simulink model "ofdm_txrx_mimo_2x"  (Simulink 6.6)
settings: Solver=VariableStepDiscrete  StartTime=0.0  StopTime=20e3  RelTol=1e-3
PreLoadFcn:
  | ofdm_rx_mimo_init
InitFcn:
  | ofdm_rx_mimo_init
StartFcn:
  | ofdm_rx_mimo_init
values from neighbouring files (symbols this model uses):
  BER_RAM_init_size = 2048    [ofdm_tx_mimo_init.m]
  CPLength = 16    [ofdm_tx_mimo_init.m]
  CRCPolynomial = hex2dec('04c11db7')    [ofdm_tx_mimo_init.m]
  freqOffset_track_filtCoef_i = hex2dec('2500')/2^32    [ofdm_rx_mimo_init.m]
  freqOffset_track_filtCoef_p = hex2dec('d0000')/2^32    [ofdm_rx_mimo_init.m]
  hi = zeros(1,length(cci))    [ofdm_rx_mimo_init.m]
  hr = zeros(1,length(ccr))    [ofdm_rx_mimo_init.m]
  max_CP_length = 16    [ofdm_tx_mimo_init.m]
  max_num_baseRateSymbols = 31    [ofdm_tx_mimo_init.m]
  max_num_subcarriers = 64    [ofdm_tx_mimo_init.m]
  max_num_trainingSymbols = 15    [ofdm_tx_mimo_init.m]
  max_numBytes = 16384    [ofdm_tx_mimo_init.m]
  max_OFDM_symbols = 2047    [ofdm_tx_mimo_init.m]
  modConstellation_qam16 = .75*[1 3 -1 -3]./3    [ofdm_tx_mimo_init.m]
  modConstellation_qam256 = 0.9375*[3 1 5 7 11 9 13 15 -3 -1 -5 -7 -11 -9 -13 -15]./15    [ofdm_tx_mimo_init.m]
  modConstellation_qam64 = 0.875*[3 1 5 7 -3 -1 -5 -7]./7    [ofdm_tx_mimo_init.m]
  modConstellation_qpsk = [1 -1]./sqrt(2)    [ofdm_tx_mimo_init.m]
  numBaseRateSymbols = 2    [ofdm_tx_mimo_init.m]
  numSubcarriers = 64    [ofdm_tx_mimo_init.m]
  numTrainingSymbols = 2    [ofdm_tx_mimo_init.m]
  pilot1_indicies = 7 + ( (64-7) * 2^16)    (= 3735559)    [ofdm_tx_mimo_init.m]
  pilot2_indicies = 21 + ( (64-21) * 2^16)    (= 2818069)    [ofdm_tx_mimo_init.m]
  pilotValue_neg = hex2dec('8000') + (2^16 * 0)    [ofdm_tx_mimo_init.m]
  pilotValue_pos = hex2dec('7FFF') + (2^16 * 0)    [ofdm_tx_mimo_init.m]
  pkt_crossCorr_thresh = 0.7    [ofdm_rx_mimo_init.m]
  pkt_energy_thresh = 0    [ofdm_rx_mimo_init.m]
  pkt_numPayloadBytes = 1400+42-6    (= 1436)    [ofdm_tx_mimo_init.m]
  pktByteNums = numHeaderBytes +  				byteIndex_numPayloadBytes(1)*2^8 +  				byteIndex_numPayloadBytes(2)*2^16 +  				byteIndex_simpleDynModMasks * 2^24    [ofdm_rx_mimo_init.m]
  PktDet_Delay = 26 + Symbol_Timing_Offset*2^7    (= 1050)    [ofdm_rx_mimo_init.m]
  preamble = 6*[0 shortsyms_10 longsyms_2]    [ofdm_tx_mimo_init.m]
  preamble_I = real(preamble)    [ofdm_tx_mimo_init.m]
  preamble_Q = imag(preamble)    [ofdm_tx_mimo_init.m]
  RAM_init_size = max_numBytes    (= 16384)    [ofdm_tx_mimo_init.m]
  reg_Interrupt_PktBuf_Control = reg_InterruptControl +  	1 * 2^16 +  	0 * 2^24    (= 65656)    [ofdm_rx_mimo_init.m]
  rx_controlBits = 1 * 2  		 + 1 * 4  		 + 0 * 8  		 + 1 * 16 * rx_SISO_Mode  		 + 1 * 32  		 + 1 * 64  		 + 0 * 128  		 + 1 * 256  		 + 0 * 512  		 + 1 * 1024  		 + 0 * 2048  		 + 1 * 4096  		 + 1 * 8192  		 + 0 * 16384  		 + 1 * 65536  		 + 1 * 131072  		 + 0 * 262144  		 + 1 * 524288    [ofdm_rx_mimo_init.m]
  Rx_PhaseNoiseTrack_K = 0.6094    [ofdm_rx_mimo_init.m]
  rx_postEq_scaling = round(rxScaling*2^11) * (1 + 2^16)    [ofdm_rx_mimo_init.m]
  RxDataScrambling_Seq = [40 198 78 63 82 173 102 245 48 111 172 115 147 230 216 93 72 65 62 2 205 242 122 90 128 83 105 97 73 10 5 252]    [ofdm_tx_mimo_init.m]
  simOnly_numSamples = length(preamble)+( (numSubcarriers+CPLength)*(numTrainingSymbols + numBaseRateSymbols + numFullRateSymbols/2) )    [ofdm_tx_mimo_init.m]
  train = [train train]    [ofdm_tx_mimo_init.m]
  tx_controlBits = (antB_preambleShift * 2^4) + (2^0 * tx_SISO_Mode) + 4*0 + 8*1    (= 73)    [ofdm_tx_mimo_init.m]
  TxDataScrambling_Seq = [40 198 78 63 82 173 102 245 48 111 172 115 147 230 216 93 72 65 62 2 205 242 122 90 128 83 105 97 73 10 5 252]    [ofdm_tx_mimo_init.m]
  txReg_symbolCounts = (2^16 * numFullRateSymbols) + (2^8 *numBaseRateSymbols ) + numTrainingSymbols    [ofdm_tx_mimo_init.m]
  TxRx_FFTScaling = bin2dec('010111') + (bin2dec('000101') * 2^6)    [ofdm_tx_mimo_init.m]
undefined symbols (not defined in the file or in its folder): AntA, AntB, BaseRate, CRC_table_gen, gcbh, intfilt, pktTiming_count_payloadStart, pktTiming_symbol_length, xlBlockLoadCallback, xlGetSimulinkPeriod
libraries: xbsIndex_r4 x3973, dspsigops x4, commchan3 x2, commrflib2 x2, dspsrcs4 x1
(part 20 of 38)

== system ofdm_txrx_mimo_2x/OFDM Tx MIMO/OutputBuffers/RAMs/Antenna A RAM ==
  I : Inport
  Q : Inport  Port=2
  We : Inport  Port=3
  Wr Addr : Inport  Port=4
  Rd Addr : Inport  Port=5
  OE# : Inport  Port=6
  Constant8 : xbsIndex_r4/Constant  arith_type=Boolean  const=0  n_bits=1  bin_pt=0  explicit_period=off  period=1  equ=P=C  opselect=C  inp2=PCIN>>17  opr=+  inp1=P  carry=CIN  dbl_ovrd=off  block_type=constant
      settings: block_version=VER_STRING_GOES_HERE
  Dual Port RAM : xbsIndex_r4/Dual Port RAM  depth=2*numSubcarriers  initVector=[repmat(0.25,1,64) repmat(0.5,1,64)]  distributed_mem=Block RAM  init_a=0  init_b=0  rst_a=off  rst_b=on  en_a=off  en_b=off  latency=1  write_mode_A=Read Before Write  write_mode_B=Read Before Write  dbl_ovrd=off  optimize=Area  use_rpm=off  block_type=dpram
      settings: xl_area=[0 0 1 0 0 0 0]  block_version=VER_STRING_GOES_HERE
  Dual Port RAM1 : xbsIndex_r4/Dual Port RAM  depth=2*numSubcarriers  initVector=[repmat(0.25,1,64) repmat(0.5,1,64)]  distributed_mem=Block RAM  init_a=0  init_b=0  rst_a=off  rst_b=on  en_a=off  en_b=off  latency=1  write_mode_A=Read Before Write  write_mode_B=Read Before Write  dbl_ovrd=off  optimize=Area  use_rpm=off  block_type=dpram
      settings: xl_area=[0 0 1 0 0 0 0]  block_version=VER_STRING_GOES_HERE
  " I" : Outport
  " Q" : Outport  Port=2
  wires:
  OE#/1 -> Dual Port RAM1/7, Dual Port RAM/7
  We/1 -> Dual Port RAM1/3, Dual Port RAM/3
  Q/1 -> Dual Port RAM1/2, Dual Port RAM1/5
  I/1 -> Dual Port RAM/2, Dual Port RAM/5
  Dual Port RAM/2 -> " I"/1
  Dual Port RAM1/2 -> " Q"/1
  Rd Addr/1 -> Dual Port RAM/4, Dual Port RAM1/4
  Constant8/1 -> Dual Port RAM1/6, Dual Port RAM/6
  Wr Addr/1 -> Dual Port RAM/1, Dual Port RAM1/1

== system ofdm_txrx_mimo_2x/OFDM Tx MIMO/OutputBuffers/RAMs/Antenna B RAM ==
  I : Inport
  Q : Inport  Port=2
  We : Inport  Port=3
  Wr Addr : Inport  Port=4
  Rd Addr : Inport  Port=5
  OE# : Inport  Port=6
  Constant8 : xbsIndex_r4/Constant  arith_type=Boolean  const=0  n_bits=1  bin_pt=0  explicit_period=off  period=1  equ=P=C  opselect=C  inp2=PCIN>>17  opr=+  inp1=P  carry=CIN  dbl_ovrd=off  block_type=constant
      settings: block_version=VER_STRING_GOES_HERE
  Dual Port RAM : xbsIndex_r4/Dual Port RAM  depth=2*numSubcarriers  initVector=-[repmat(0.25,1,64) repmat(0.5,1,64)]  distributed_mem=Block RAM  init_a=0  init_b=0  rst_a=off  rst_b=on  en_a=off  en_b=off  latency=1  write_mode_A=Read Before Write  write_mode_B=Read Before Write  dbl_ovrd=off  optimize=Area  use_rpm=off  block_type=dpram
      settings: xl_area=[0 0 1 0 0 0 0]  block_version=VER_STRING_GOES_HERE
  Dual Port RAM1 : xbsIndex_r4/Dual Port RAM  depth=2*numSubcarriers  initVector=-[repmat(0.25,1,64) repmat(0.5,1,64)]  distributed_mem=Block RAM  init_a=0  init_b=0  rst_a=off  rst_b=on  en_a=off  en_b=off  latency=1  write_mode_A=Read Before Write  write_mode_B=Read Before Write  dbl_ovrd=off  optimize=Area  use_rpm=off  block_type=dpram
      settings: xl_area=[0 0 1 0 0 0 0]  block_version=VER_STRING_GOES_HERE
  " I" : Outport
  " Q" : Outport  Port=2
  wires:
  Wr Addr/1 -> Dual Port RAM1/1, Dual Port RAM/1
  Constant8/1 -> Dual Port RAM/6, Dual Port RAM1/6
  Rd Addr/1 -> Dual Port RAM1/4, Dual Port RAM/4
  Dual Port RAM1/2 -> " Q"/1
  Dual Port RAM/2 -> " I"/1
  I/1 -> Dual Port RAM/5, Dual Port RAM/2
  Q/1 -> Dual Port RAM1/5, Dual Port RAM1/2
  We/1 -> Dual Port RAM1/3, Dual Port RAM/3
  OE#/1 -> Dual Port RAM/7, Dual Port RAM1/7

== system ofdm_txrx_mimo_2x/OFDM Tx MIMO/OutputMuxes/Preamble Generation/2x ==
  I : Inport
  Concat1 : xbsIndex_r4/Concat  num_inputs=2  dbl_ovrd=off  block_type=concat
      settings: block_version=VER_STRING_GOES_HERE
  Constant : xbsIndex_r4/Constant  arith_type=Unsigned  const=0  n_bits=1  bin_pt=0  explicit_period=off  period=1  equ=P=C  opselect=C  inp2=PCIN>>17  opr=+  inp1=P  carry=CIN  dbl_ovrd=off  block_type=constant
      settings: block_version=VER_STRING_GOES_HERE
  O : Outport
  wires:
  Constant/1 -> Concat1/2
  I/1 -> Concat1/1
  Concat1/1 -> O/1

== system ofdm_txrx_mimo_2x/OFDM Tx MIMO/OutputMuxes/Preamble Generation/2x1 ==
  I : Inport
  Concat1 : xbsIndex_r4/Concat  num_inputs=2  dbl_ovrd=off  block_type=concat
      settings: block_version=VER_STRING_GOES_HERE
  Constant : xbsIndex_r4/Constant  arith_type=Unsigned  const=0  n_bits=1  bin_pt=0  explicit_period=off  period=1  equ=P=C  opselect=C  inp2=PCIN>>17  opr=+  inp1=P  carry=CIN  dbl_ovrd=off  block_type=constant
      settings: block_version=VER_STRING_GOES_HERE
  O : Outport
  wires:
  Concat1/1 -> O/1
  I/1 -> Concat1/1
  Constant/1 -> Concat1/2

== system ofdm_txrx_mimo_2x/OFDM Tx MIMO/OutputMuxes/Preamble Generation/2x2 ==
  I : Inport
  Concat1 : xbsIndex_r4/Concat  num_inputs=2  dbl_ovrd=off  block_type=concat
      settings: block_version=VER_STRING_GOES_HERE
  Constant : xbsIndex_r4/Constant  arith_type=Unsigned  const=0  n_bits=1  bin_pt=0  explicit_period=off  period=1  equ=P=C  opselect=C  inp2=PCIN>>17  opr=+  inp1=P  carry=CIN  dbl_ovrd=off  block_type=constant
      settings: block_version=VER_STRING_GOES_HERE
  O : Outport
  wires:
  Constant/1 -> Concat1/2
  I/1 -> Concat1/1
  Concat1/1 -> O/1

== system ofdm_txrx_mimo_2x/OFDM Tx MIMO/OutputMuxes/Preamble Generation/2x3 ==
  I : Inport
  Concat1 : xbsIndex_r4/Concat  num_inputs=2  dbl_ovrd=off  block_type=concat
      settings: block_version=VER_STRING_GOES_HERE
  Constant : xbsIndex_r4/Constant  arith_type=Unsigned  const=0  n_bits=1  bin_pt=0  explicit_period=off  period=1  equ=P=C  opselect=C  inp2=PCIN>>17  opr=+  inp1=P  carry=CIN  dbl_ovrd=off  block_type=constant
      settings: block_version=VER_STRING_GOES_HERE
  O : Outport
  wires:
  Concat1/1 -> O/1
  I/1 -> Concat1/1
  Constant/1 -> Concat1/2

== system ofdm_txrx_mimo_2x/OFDM Tx MIMO/OutputMuxes/Preamble Generation/A btwn B&C ==
  A : Inport
  B : Inport  Port=2
  C : Inport  Port=3
  Logical1 : xbsIndex_r4/Logical  logical_function=AND  inputs=2  en=off  latency=0  precision=Full  arith_type=Unsigned  n_bits=8  bin_pt=2  align_bp=on  dbl_ovrd=off  block_type=logical
      settings: xl_area=[1 0 0 1 0 0 0]  block_version=VER_STRING_GOES_HERE
  Relational1 : xbsIndex_r4/Relational  mode=a<=b  en=off  latency=0  dbl_ovrd=off  block_type=relational
      settings: xl_area=[3 0 0 5 0 0 0]  block_version=VER_STRING_GOES_HERE
  Relational5 : xbsIndex_r4/Relational  mode=a>b  en=off  latency=0  dbl_ovrd=off  block_type=relational
      settings: xl_area=[3 0 0 5 0 0 0]  block_version=VER_STRING_GOES_HERE
  Q : Outport
  wires:
  Relational5/1 -> Logical1/1
  Relational1/1 -> Logical1/2
  A/1 -> Relational5/1, Relational1/1
  B/1 -> Relational5/2
  Logical1/1 -> Q/1
  C/1 -> Relational1/2

== system ofdm_txrx_mimo_2x/OFDM Tx MIMO/OutputMuxes/Preamble Generation/A btwn B&C1 ==
  A : Inport
  B : Inport  Port=2
  C : Inport  Port=3
  Logical1 : xbsIndex_r4/Logical  logical_function=AND  inputs=2  en=off  latency=0  precision=Full  arith_type=Unsigned  n_bits=8  bin_pt=2  align_bp=on  dbl_ovrd=off  block_type=logical
      settings: xl_area=[1 0 0 1 0 0 0]  block_version=VER_STRING_GOES_HERE
  Relational1 : xbsIndex_r4/Relational  mode=a<=b  en=off  latency=0  dbl_ovrd=off  block_type=relational
      settings: xl_area=[3 0 0 5 0 0 0]  block_version=VER_STRING_GOES_HERE
  Relational5 : xbsIndex_r4/Relational  mode=a>b  en=off  latency=0  dbl_ovrd=off  block_type=relational
      settings: xl_area=[3 0 0 5 0 0 0]  block_version=VER_STRING_GOES_HERE
  Q : Outport
  wires:
  C/1 -> Relational1/2
  Logical1/1 -> Q/1
  B/1 -> Relational5/2
  A/1 -> Relational1/1, Relational5/1
  Relational1/1 -> Logical1/2
  Relational5/1 -> Logical1/1

== system ofdm_txrx_mimo_2x/OFDM Tx MIMO/OutputMuxes/Preamble Generation/Ant B Addr ==
  A-Addr : Inport
  AddSub : xbsIndex_r4/AddSub  mode=Addition  use_carryin=off  use_carryout=off  en=off  latency=0  precision=User Defined  arith_type=Unsigned  n_bits=ceil(log2(length(preamble_I)))  bin_pt=0  quantization=Truncate  overflow=Wrap  dbl_ovrd=off  use_behavioral_HDL=off  pipelined=off  use_rpm=on  hw_selection=Fabric  block_type=addsub
      settings: block_version=8.2.02
  AddSub1 : xbsIndex_r4/AddSub  mode=Subtraction  use_carryin=off  use_carryout=off  en=off  latency=0  precision=User Defined  arith_type=Unsigned  n_bits=ceil(log2(length(preamble_I)))  bin_pt=0  quantization=Truncate  overflow=Wrap  dbl_ovrd=off  use_behavioral_HDL=off  pipelined=off  use_rpm=on  hw_selection=Fabric  block_type=addsub
      settings: block_version=8.2.02
  Constant : xbsIndex_r4/Constant  arith_type=Unsigned  const=length(preamble_I)  n_bits=ceil(log2(length(preamble_I)))  bin_pt=0  explicit_period=off  period=1  equ=P=C  opselect=C  inp2=PCIN>>17  opr=+  inp1=P  carry=CIN  dbl_ovrd=off  block_type=constant
      settings: block_version=8.2.02
  Down Sample1 : xbsIndex_r4/Down Sample  sample_ratio=2  sample_phase="Last Value of Frame  (most efficient)"  en=off  latency=1  dbl_ovrd=off  block_type=dsamp
      settings: block_version=VER_STRING_GOES_HERE
  Mux : xbsIndex_r4/Mux  inputs=2  en=off  latency=0  precision=Full  arith_type=Unsigned  n_bits=16  bin_pt=14  quantization=Truncate  overflow=Wrap  dbl_ovrd=off  block_type=mux
      settings: block_version=8.2.02
  Relational : xbsIndex_r4/Relational  mode=a<=b  en=off  latency=0  dbl_ovrd=off  block_type=relational
      settings: block_version=8.2.02
  Shifted Preamble : Scope  NumInputPorts=4
      settings: TimeRange=4000  YMin=-0.8~-0.8~-1~0  YMax=1~0.8~1~65  SaveName=ScopeData64
  done{3..6} (4 alike) : xbsIndex_r4/Gateway Out  hdl_port=off  timing_constraint=None  locs_specified=off  LOCs={}  block_type=gatewayout
      settings: infoedit="Gateway out block.  Converts Xilinx fixed point inputs into ouputs of type Simulink integer, double, or fixed point.<P><P>Hardware notes:  In hardware these blocks become top level output ports or are discarded, depending on how they are configured."  xl_area=[0 0 0 0 1 0 0]  block_version=VER_STRING_GOES_HERE
  B-Addr : Outport
  wires:
  AddSub/1 -> Mux/2, Relational/2, done5/1, AddSub1/1
  Constant/1 -> Relational/1, AddSub1/2
  A-Addr/1 -> AddSub/1, done4/1
  [TxReg_AntBPreambleShift] -> Down Sample1/1
  Relational/1 -> Mux/1, done3/1
  AddSub1/1 -> Mux/3
  done4/1 -> Shifted Preamble/1
  done5/1 -> Shifted Preamble/2
  done3/1 -> Shifted Preamble/3
  done6/1 -> Shifted Preamble/4
  Mux/1 -> done6/1, B-Addr/1
  Down Sample1/1 -> AddSub/2

== system ofdm_txrx_mimo_2x/OFDM Tx MIMO/Training_Data/Ant Selector/Oscillator ==
  rst : Inport
  en : Inport  Port=2
  Assert : xbsIndex_r4/Assert  Orientation=left  assert_type=on  type_source=Explicitly  arith_type=Boolean  n_bits=16  bin_pt=14  assert_rate=on  rate_source=Explicitly  period=2  output_port=on  block_type=assert
      settings: infoedit=Asserts a user-defined sample rate and/or type on a signal.<P><P>Hardware notes: In hardware this block costs nothing.  block_version=VER_STRING_GOES_HERE
  Inverter1 : xbsIndex_r4/Inverter  Orientation=left  en=off  latency=0  dbl_ovrd=off  block_type=inv
      settings: block_version=VER_STRING_GOES_HERE
  Register : xbsIndex_r4/Register  init=0  rst=on  en=on  dbl_ovrd=off  block_type=register
      settings: block_version=VER_STRING_GOES_HERE
  Q : Outport
  wires:
  en/1 -> Register/3
  Inverter1/1 -> Register/1
  Assert/1 -> Inverter1/1
  Register/1 -> Assert/1, Q/1
  rst/1 -> Register/2

== system ofdm_txrx_mimo_2x/OFDM Tx MIMO/Training_Data/Ant Selector/negedge ==
  D : Inport
  Delay : xbsIndex_r4/Delay  en=off  latency=1  dbl_ovrd=off  reg_retiming=on  block_type=delay
      settings: infoedit=Hardware notes: A delay line is a chain, each link of which is an SRL16 followed by a flip-flop. If register retiming is enabled, the delay line is a chain of flip-flops.  block_version=VER_STRING_GOES_HERE
  Inverter1 : xbsIndex_r4/Inverter  en=off  latency=0  dbl_ovrd=off  block_type=inv
      settings: block_version=VER_STRING_GOES_HERE
  Logical3 : xbsIndex_r4/Logical  logical_function=AND  inputs=2  en=off  latency=0  precision=Full  arith_type=Unsigned  n_bits=16  bin_pt=0  align_bp=on  dbl_ovrd=off  block_type=logical
      settings: block_version=VER_STRING_GOES_HERE
  Q : Outport
  wires:
  Inverter1/1 -> Logical3/1
  D/1 -> Inverter1/1, Delay/1
  Delay/1 -> Logical3/2
  Logical3/1 -> Q/1

== system ofdm_txrx_mimo_2x/OFDM Tx MIMO/Training_Data/FlexibleMod/DataModulation ==
  note: Latency = 1
  note: This system allows for the packet to be loaded into the RAM and the read into the system which turns it into a modulated signal with varying modulation types. The output of the system is the I and Q values of the signal.
      | This block will not begin outputting data until the ready bit has been asserted.  Therefore, the ready bit should be asserted only once a sufficient number of bytes have been loaded into the RAM so
      |  that this block will not try to take information out of the RAM that is not there yet.  When ready is asserted the length of the packet should be on the input length.  Sub sync will be asserted by the IFFT block in order to
      | insure that each subcarrier will use the desired number of bits.  Sub sync should be asserted 2 sample periods before the IFFT block expects the 0th subcarrier's I and Q information to come from this block.
      | The done output is a pulse that indicates the entire packet has been sent.
  note: Latency = 1
  note: Latency = 0
  ready : Inport
  ifft_index : Inport  Port=2
  valid : Inport  Port=3
  BaseRate_En : Inport  Port=4
  ant_sel : Inport  Port=5
  PktBuffer_CRC1 : Subsystem  Orientation=left
  QAM : Subsystem
  Tx Pkt Buf1 : Scope  NumInputPorts=5
      settings: TimeRange=5000  YMin=0~0~0~0~-1  YMax=130~120~1~1~1  SaveName=ScopeData2
  control : Subsystem
  data_buffer : Subsystem
  done{1,3..6} (5 alike) : xbsIndex_r4/Gateway Out  hdl_port=off  timing_constraint=None  locs_specified=off  LOCs={}  block_type=gatewayout
      settings: infoedit="Gateway out block.  Converts Xilinx fixed point inputs into ouputs of type Simulink integer, double, or fixed point.<P><P>Hardware notes:  In hardware these blocks become top level output ports or are discarded, depending on how they are configured."  xl_area=[0 0 0 0 1 0 0]  block_version=VER_STRING_GOES_HERE
  slicer : Subsystem
  Q : Outport
  I : Outport  Port=2
  wires:
  control/4(mod) -> data_buffer/4(mod), QAM/1(Mod Sel), done4/1
  valid/1 -> control/6(valid)
  control/3(index) -> data_buffer/3(index), slicer/2(index)
  slicer/1(Byte Out) -> QAM/2(Mod Bits), done1/1
  QAM/1(I) -> I/1, done3/1
  QAM/2(Q) -> Q/1, done5/1
  data_buffer/3(16-Bit Register) -> slicer/1(16-Bit In), done6/1
  control/2(reset) -> data_buffer/2(reset)
  ifft_index/1 -> control/4(ifft_index)
  BaseRate_En/1 -> control/5(BaseRate_En)
  ant_sel/1 -> control/7(ant_sel)
  done1/1 -> Tx Pkt Buf1/2
  done3/1 -> Tx Pkt Buf1/3
  done4/1 -> Tx Pkt Buf1/1
  done5/1 -> Tx Pkt Buf1/4
  done6/1 -> Tx Pkt Buf1/5
  ready/1 -> control/3(ready), PktBuffer_CRC1/1(Rst)
  PktBuffer_CRC1/1(NumBytes) -> control/2(length)
  PktBuffer_CRC1/2(FullRateMasks) -> control/1(FullRateMasks)
  PktBuffer_CRC1/3(Dout) -> data_buffer/1(RAM data)
  data_buffer/1(RAM address (B)) -> PktBuffer_CRC1/3(DataOut_Addr)
  data_buffer/2(RAM addr Vout) -> PktBuffer_CRC1/2(DataOut_Addr Vin)

== system ofdm_txrx_mimo_2x/OFDM Tx MIMO/Training_Data/FlexibleMod/S-R Latch ==
  R : Inport
  S : Inport  Port=2
  Constant3 : xbsIndex_r4/Constant  arith_type=Boolean  const=1  n_bits=1  bin_pt=0  explicit_period=off  period=1  equ=P=C  opselect=C  inp2=PCIN>>17  opr=+  inp1=P  carry=CIN  dbl_ovrd=off  block_type=constant
      settings: block_version=VER_STRING_GOES_HERE
  Register : xbsIndex_r4/Register  init=0  rst=on  en=on  dbl_ovrd=off  block_type=register
      settings: xl_area=[0, 0, 0, 0, 0, 0, 0]  block_version=VER_STRING_GOES_HERE
  Q : Outport
  wires:
  Constant3/1 -> Register/1
  R/1 -> Register/2
  Register/1 -> Q/1
  S/1 -> Register/3

== system ofdm_txrx_mimo_2x/OFDM Tx MIMO/Training_Data/FlexibleMod/posedge ==
  signal : Inport
  Delay3 : Subsystem
  Inverter2 : Subsystem
  Logical3 : Subsystem
  pulse : Outport
  wires:
  Inverter2/1(out0) -> Delay3/1(in0)
  signal/1 -> Inverter2/1(in0), Logical3/1(in0)
  Delay3/1(out0) -> Logical3/2(in1)
  Logical3/1(out0) -> pulse/1

== system ofdm_txrx_mimo_2x/OFDM Tx MIMO/Training_Data/Training_Pilots/Scrambled_Pilot_Inserter ==
  note: The IFFT outputs xn_index three cycles before it expects
      | a given input sample. The index must be delayed here
      | to account for that.
  note: No data should be placed
      | on the subcarrier at DC (xn=0)
  note: FIXME: How should pilot scrambling work with 2 transmit antennas?
      | It's currently configured to treat each new OFDM symbol independently,
      | scrambling the antennas' pilots independently. Check 802.11n...?
  IFFT Index : Inport
  "I " : Inport  Port=2
  "Q " : Inport  Port=3
  rst : Inport  Port=4
  Ant Sel : Inport  Port=5
  16LSB : xbsIndex_r4/Slice  nbits=16  boolean_output=off  mode=Lower Bit Location + Width  bit1=0  base1=MSB of Input  bit0=0  base0=LSB of Input  dbl_ovrd=off  block_type=slice
      settings: infoedit="Extracts a given range of bits from each input sample and presents it at the output.  The output type is ordinarily unsigned with binary point at zero, but can be Boolean when the slice is one bit wide.<P><P>Hardware notes: In hardware this block costs nothing."  block_version=VER_STRING_GOES_HERE
  16LSB1 : xbsIndex_r4/Slice  nbits=16  boolean_output=off  mode=Lower Bit Location + Width  bit1=0  base1=MSB of Input  bit0=0  base0=LSB of Input  dbl_ovrd=off  block_type=slice
      settings: infoedit="Extracts a given range of bits from each input sample and presents it at the output.  The output type is ordinarily unsigned with binary point at zero, but can be Boolean when the slice is one bit wide.<P><P>Hardware notes: In hardware this block costs nothing."  block_version=VER_STRING_GOES_HERE
  16MSB : xbsIndex_r4/Slice  nbits=16  boolean_output=off  mode=Lower Bit Location + Width  bit1=0  base1=MSB of Input  bit0=16  base0=LSB of Input  dbl_ovrd=off  block_type=slice
      settings: infoedit="Extracts a given range of bits from each input sample and presents it at the output.  The output type is ordinarily unsigned with binary point at zero, but can be Boolean when the slice is one bit wide.<P><P>Hardware notes: In hardware this block costs nothing."  block_version=VER_STRING_GOES_HERE
  16MSB1 : xbsIndex_r4/Slice  nbits=16  boolean_output=off  mode=Lower Bit Location + Width  bit1=0  base1=MSB of Input  bit0=16  base0=LSB of Input  dbl_ovrd=off  block_type=slice
      settings: infoedit="Extracts a given range of bits from each input sample and presents it at the output.  The output type is ordinarily unsigned with binary point at zero, but can be Boolean when the slice is one bit wide.<P><P>Hardware notes: In hardware this block costs nothing."  block_version=VER_STRING_GOES_HERE
  6LSB : xbsIndex_r4/Slice  nbits=ceil(log2(numSubcarriers))  boolean_output=off  mode=Lower Bit Location + Width  bit1=0  base1=MSB of Input  bit0=0  base0=LSB of Input  dbl_ovrd=off  block_type=slice
      settings: infoedit="Extracts a given range of bits from each input sample and presents it at the output.  The output type is ordinarily unsigned with binary point at zero, but can be Boolean when the slice is one bit wide.<P><P>Hardware notes: In hardware this block costs nothing."  block_version=VER_STRING_GOES_HERE
  6LSB+16 : xbsIndex_r4/Slice  nbits=ceil(log2(numSubcarriers))  boolean_output=off  mode=Lower Bit Location + Width  bit1=0  base1=MSB of Input  bit0=16  base0=LSB of Input  dbl_ovrd=off  block_type=slice
      settings: infoedit="Extracts a given range of bits from each input sample and presents it at the output.  The output type is ordinarily unsigned with binary point at zero, but can be Boolean when the slice is one bit wide.<P><P>Hardware notes: In hardware this block costs nothing."  block_version=VER_STRING_GOES_HERE
  6LSB+16_ : xbsIndex_r4/Slice  nbits=ceil(log2(numSubcarriers))  boolean_output=off  mode=Lower Bit Location + Width  bit1=0  base1=MSB of Input  bit0=16  base0=LSB of Input  dbl_ovrd=off  block_type=slice
      settings: infoedit="Extracts a given range of bits from each input sample and presents it at the output.  The output type is ordinarily unsigned with binary point at zero, but can be Boolean when the slice is one bit wide.<P><P>Hardware notes: In hardware this block costs nothing."  block_version=VER_STRING_GOES_HERE
  6LSB_ : xbsIndex_r4/Slice  nbits=ceil(log2(numSubcarriers))  boolean_output=off  mode=Lower Bit Location + Width  bit1=0  base1=MSB of Input  bit0=0  base0=LSB of Input  dbl_ovrd=off  block_type=slice
      settings: infoedit="Extracts a given range of bits from each input sample and presents it at the output.  The output type is ordinarily unsigned with binary point at zero, but can be Boolean when the slice is one bit wide.<P><P>Hardware notes: In hardware this block costs nothing."  block_version=VER_STRING_GOES_HERE
  Concat1 : xbsIndex_r4/Concat  num_inputs=2  dbl_ovrd=off  block_type=concat
      settings: block_version=VER_STRING_GOES_HERE
  Constant2 : xbsIndex_r4/Constant  arith_type=Signed (2's comp)  const=0  n_bits=1  bin_pt=0  explicit_period=off  period=1  equ=P=C  opselect=C  inp2=PCIN>>17  opr=+  inp1=P  carry=CIN  dbl_ovrd=off  block_type=constant
      settings: block_version=VER_STRING_GOES_HERE
  Constant4 : xbsIndex_r4/Constant  arith_type=Unsigned  const=0  n_bits=1  bin_pt=0  explicit_period=off  period=2  equ=P=C  opselect=C  inp2=PCIN>>17  opr=+  inp1=P  carry=CIN  dbl_ovrd=off  block_type=constant
      settings: block_version=VER_STRING_GOES_HERE
  Constant5 : xbsIndex_r4/Constant  arith_type=Unsigned  const=63  n_bits=6  bin_pt=0  explicit_period=off  period=2  equ=P=C  opselect=C  inp2=PCIN>>17  opr=+  inp1=P  carry=CIN  dbl_ovrd=off  block_type=constant
      settings: block_version=VER_STRING_GOES_HERE
  Convert : xbsIndex_r4/Convert  arith_type=Unsigned  n_bits=1  bin_pt=0  quantization=Truncate  overflow=Wrap  latency=0  dbl_ovrd=off  pipeline=off  block_type=convert
      settings: xl_area=[0, 0, 0, 0, 0, 0, 0]  block_version=VER_STRING_GOES_HERE
  Convert1 : xbsIndex_r4/Convert  arith_type=Unsigned  n_bits=1  bin_pt=0  quantization=Truncate  overflow=Wrap  latency=0  dbl_ovrd=off  pipeline=off  block_type=convert
      settings: xl_area=[0, 0, 0, 0, 0, 0, 0]  block_version=VER_STRING_GOES_HERE
  Delay : xbsIndex_r4/Delay  en=off  latency=3  dbl_ovrd=off  reg_retiming=off  block_type=delay
      settings: infoedit=Hardware notes: A delay line is a chain, each link of which is an SRL16 followed by a flip-flop. If register retiming is enabled, the delay line is a chain of flip-flops.  xl_area=[3 6 0 6 0 0 0]  block_version=VER_STRING_GOES_HERE
  Down Sample3 : xbsIndex_r4/Down Sample  sample_ratio=2  sample_phase="Last Value of Frame  (most efficient)"  en=off  latency=1  dbl_ovrd=off  block_type=dsamp
      settings: block_version=VER_STRING_GOES_HERE
  Down Sample4 : xbsIndex_r4/Down Sample  sample_ratio=2  sample_phase="Last Value of Frame  (most efficient)"  en=off  latency=1  dbl_ovrd=off  block_type=dsamp
      settings: block_version=VER_STRING_GOES_HERE
  Gateway Out{,1..4} (5 alike) : xbsIndex_r4/Gateway Out  hdl_port=off  timing_constraint=None  locs_specified=off  LOCs={}  block_type=gatewayout
      settings: infoedit="Gateway out block.  Converts Xilinx fixed point inputs into ouputs of type Simulink integer, double, or fixed point.<P><P>Hardware notes:  In hardware these blocks become top level output ports or are discarded, depending on how they are configured."  xl_area=[0, 0, 0, 0, 0, 0, 0]  block_version=VER_STRING_GOES_HERE
  Inverter : xbsIndex_r4/Inverter  Orientation=up  en=off  latency=0  dbl_ovrd=off  block_type=inv
      settings: block_version=VER_STRING_GOES_HERE
  Logical : xbsIndex_r4/Logical  logical_function=OR  inputs=2  en=off  latency=0  precision=Full  arith_type=Unsigned  n_bits=8  bin_pt=2  align_bp=on  dbl_ovrd=off  block_type=logical
      settings: xl_area=[2 0 0 2 0 0 0]  block_version=VER_STRING_GOES_HERE
  Logical1 : xbsIndex_r4/Logical  logical_function=OR  inputs=3  en=off  latency=0  precision=Full  arith_type=Unsigned  n_bits=8  bin_pt=2  align_bp=on  dbl_ovrd=off  block_type=logical
      settings: xl_area=[2 0 0 2 0 0 0]  block_version=VER_STRING_GOES_HERE
  Logical2 : xbsIndex_r4/Logical  logical_function=AND  inputs=2  en=off  latency=0  precision=Full  arith_type=Unsigned  n_bits=8  bin_pt=2  align_bp=on  dbl_ovrd=off  block_type=logical
      settings: xl_area=[2 0 0 2 0 0 0]  block_version=VER_STRING_GOES_HERE
  Mux : xbsIndex_r4/Mux  inputs=4  en=off  latency=0  precision=Full  arith_type="Signed  (2's comp)"  n_bits=8  bin_pt=2  quantization=Truncate  overflow=Wrap  dbl_ovrd=off  block_type=mux
      settings: xl_area=[16 0 0 32 0 0 0]  block_version=VER_STRING_GOES_HERE
  Mux1 : xbsIndex_r4/Mux  inputs=4  en=off  latency=0  precision=Full  arith_type="Signed  (2's comp)"  n_bits=8  bin_pt=2  quantization=Truncate  overflow=Wrap  dbl_ovrd=off  block_type=mux
      settings: xl_area=[16 0 0 32 0 0 0]  block_version=VER_STRING_GOES_HERE
  Mux2 : xbsIndex_r4/Mux  inputs=2  en=off  latency=0  precision=Full  arith_type="Signed  (2's comp)"  n_bits=8  bin_pt=2  quantization=Truncate  overflow=Wrap  dbl_ovrd=off  block_type=mux
      settings: xl_area=[16 0 0 32 0 0 0]  block_version=VER_STRING_GOES_HERE
  Mux3 : xbsIndex_r4/Mux  inputs=2  en=off  latency=0  precision=Full  arith_type="Signed  (2's comp)"  n_bits=8  bin_pt=2  quantization=Truncate  overflow=Wrap  dbl_ovrd=off  block_type=mux
      settings: xl_area=[16 0 0 32 0 0 0]  block_version=VER_STRING_GOES_HERE
  Pilot Scrambling Sequence Gen : Subsystem
  Reinterpret{,1..3} (4 alike) : xbsIndex_r4/Reinterpret  force_arith_type=on  arith_type="Signed  (2's comp)"  force_bin_pt=on  bin_pt=15  block_type=cast
      settings: infoedit="Changes type of samples without altering their binary representation.<P><P>Hardware notes: In hardware this block costs nothing.<P><P>Example:  Suppose the input is 6 bits wide, signed, with 2 fractional bits, and the output is forced to unsigned with 0 fractional bits.  Then an input of -2.0 (1110.00 in binary 2's complement) becomes an output of 56 (111000 in binary)."  block_version=VER_STRING_GOES_HERE
  Relational{1..2,4..7} (6 alike) : xbsIndex_r4/Relational  mode=a=b  en=off  latency=0  dbl_ovrd=off  block_type=relational
      Relational1 settings: xl_area=[6 0 0 11 0 0 0]  block_version=VER_STRING_GOES_HERE
      Relational2 settings: xl_area=[6 0 0 11 0 0 0]  block_version=VER_STRING_GOES_HERE
      Relational4 settings: xl_area=[2 0 0 3 0 0 0]  block_version=VER_STRING_GOES_HERE
      Relational5 settings: xl_area=[2 0 0 3 0 0 0]  block_version=VER_STRING_GOES_HERE
      Relational6 settings: xl_area=[2 0 0 3 0 0 0]  block_version=VER_STRING_GOES_HERE
      Relational7 settings: xl_area=[2 0 0 3 0 0 0]  block_version=VER_STRING_GOES_HERE
  Some Logic : Subsystem
  Tx Pilots : Scope  NumInputPorts=5
      settings: YMin=0~0~0~-1~-1  YMax=65~1~3~1~1
  " I" : Outport
  " Q" : Outport  Port=2
  wires:
  "I "/1 -> Mux/2
  Mux/1 -> " I"/1, Gateway Out3/1
  "Q "/1 -> Mux1/2
  Constant2/1 -> Mux1/3, Mux/5, Mux1/4, Mux1/5
  Mux1/1 -> " Q"/1, Gateway Out4/1
  Constant4/1 -> Relational1/1
  Relational1/1 -> Concat1/1, Concat1/2
  Concat1/1 -> Logical/1
  Logical/1 -> Mux1/1, Mux/1, Gateway Out2/1
  6LSB_/1 -> Relational6/1
  6LSB+16_/1 -> Relational7/1
  6LSB+16/1 -> Relational5/1
  6LSB/1 -> Relational4/1
  IFFT Index/1 -> Delay/1
  Delay/1 -> Relational5/2, Relational7/2, Relational6/2, Relational4/2, Relational1/2, Gateway Out/1, Relational2/2
  Relational6/1 -> Convert1/1
  Mux3/1 -> Down Sample4/1
  Mux2/1 -> Down Sample3/1
  Logical1/1 -> Convert/1
  Relational4/1 -> Logical1/1
  Relational5/1 -> Logical1/2
  Relational7/1 -> Logical1/3
  rst/1 -> Pilot Scrambling Sequence Gen/1(rst)
  Gateway Out/1 -> Tx Pilots/1
  Gateway Out1/1 -> Tx Pilots/2
  Gateway Out2/1 -> Tx Pilots/3
  Gateway Out3/1 -> Tx Pilots/4
  Gateway Out4/1 -> Tx Pilots/5
  Constant5/1 -> Relational2/1
  Pilot Scrambling Sequence Gen/1(Q) -> Some Logic/1(z), Gateway Out1/1
  Some Logic/1(Q) -> Logical/2
  Convert/1 -> Some Logic/2(x)
  Convert1/1 -> Some Logic/3(y)
  [regTxRx_PilotToneIndex1] -> 6LSB+16/1, 6LSB/1
  [regTxRx_PilotToneIndex2] -> 6LSB_/1, 6LSB+16_/1
  Down Sample3/1 -> Mux/3
  Down Sample4/1 -> Mux/4
  [regTx_PilotToneValue2] -> 16LSB1/1, 16MSB1/1
  [regTx_PilotToneValue1] -> 16LSB/1, 16MSB/1
  16LSB/1 -> Reinterpret/1
  16MSB/1 -> Reinterpret1/1
  16LSB1/1 -> Reinterpret2/1
  16MSB1/1 -> Reinterpret3/1
  Reinterpret/1 -> Mux2/2
  Reinterpret1/1 -> Mux2/3
  Reinterpret2/1 -> Mux3/2
  Reinterpret3/1 -> Mux3/3
  Ant Sel/1 -> Mux2/1, Mux3/1, Inverter/1
  Logical2/1 -> Pilot Scrambling Sequence Gen/2(en)
  Relational2/1 -> Logical2/1
  Inverter/1 -> Logical2/2

== system ofdm_txrx_mimo_2x/OFDM Tx MIMO/TxControl/Start/Done Control/Posedge2 ==
  D : Inport
  Delay : xbsIndex_r4/Delay  en=off  latency=1  dbl_ovrd=off  reg_retiming=off  block_type=delay
      settings: infoedit=Hardware notes: A delay line is a chain, each link of which is an SRL16 followed by a flip-flop. If register retiming is enabled, the delay line is a chain of flip-flops.  xl_area=[1 1 0 0 0 0 0]  block_version=VER_STRING_GOES_HERE
  Inverter : xbsIndex_r4/Inverter  en=off  latency=0  dbl_ovrd=off  block_type=inv
      settings: xl_area=[0 0 0 0 0 0 0]  block_version=VER_STRING_GOES_HERE
  Logical : xbsIndex_r4/Logical  logical_function=AND  inputs=2  en=off  latency=0  precision=Full  arith_type=Unsigned  n_bits=1  bin_pt=0  align_bp=on  dbl_ovrd=off  block_type=logical
      settings: xl_area=[1 0 0 1 0 0 0]  block_version=VER_STRING_GOES_HERE
  Q : Outport
  wires:
  Delay/1 -> Inverter/1
  Inverter/1 -> Logical/2
  D/1 -> Logical/1, Delay/1
  Logical/1 -> Q/1

== system ofdm_txrx_mimo_2x/OFDM Tx MIMO/TxControl/Start/Done Control/Posedge3 ==
  D : Inport
  Delay : xbsIndex_r4/Delay  en=off  latency=1  dbl_ovrd=off  reg_retiming=off  block_type=delay
      settings: infoedit=Hardware notes: A delay line is a chain, each link of which is an SRL16 followed by a flip-flop. If register retiming is enabled, the delay line is a chain of flip-flops.  xl_area=[1 1 0 0 0 0 0]  block_version=VER_STRING_GOES_HERE
  Inverter : xbsIndex_r4/Inverter  en=off  latency=0  dbl_ovrd=off  block_type=inv
      settings: xl_area=[0 0 0 0 0 0 0]  block_version=VER_STRING_GOES_HERE
  Logical : xbsIndex_r4/Logical  logical_function=AND  inputs=2  en=off  latency=0  precision=Full  arith_type=Unsigned  n_bits=1  bin_pt=0  align_bp=on  dbl_ovrd=off  block_type=logical
      settings: xl_area=[1 0 0 1 0 0 0]  block_version=VER_STRING_GOES_HERE
  Q : Outport
  wires:
  Logical/1 -> Q/1
  D/1 -> Delay/1, Logical/1
  Inverter/1 -> Logical/2
  Delay/1 -> Inverter/1

== system ofdm_txrx_mimo_2x/OFDM Tx MIMO/TxControl/Start/Done Control/S-R Latch ==
  S : Inport
  R : Inport  Port=2
  Constant2 : xbsIndex_r4/Constant  arith_type=Unsigned  const=1  n_bits=1  bin_pt=0  explicit_period=off  period=1  equ=P=C  opselect=C  inp2=PCIN>>17  opr=+  inp1=P  carry=CIN  dbl_ovrd=off  block_type=constant
      settings: block_version=VER_STRING_GOES_HERE
  Reg : xbsIndex_r4/Register  init=0  rst=on  en=on  dbl_ovrd=off  block_type=register
      settings: xl_area=[1 1 0 0 0 0 0]  block_version=VER_STRING_GOES_HERE
  Q : Outport
  wires:
  Constant2/1 -> Reg/1
  R/1 -> Reg/2
  Reg/1 -> Q/1
  S/1 -> Reg/3

== system ofdm_txrx_mimo_2x/OFDM Rx MIMO/ADC Inputs & Antenna Selection/Antenna Selection/AntA_Gains/Posedge ==
  note: Rising Edge Detection
  D : Inport
  Delay : xbsIndex_r4/Delay  en=off  latency=1  dbl_ovrd=off  reg_retiming=off  block_type=delay
      settings: infoedit=Hardware notes: A delay line is a chain, each link of which is an SRL16 followed by a flip-flop. If register retiming is enabled, the delay line is a chain of flip-flops.  xl_area=[1 1 0 0 0 0 0]  block_version=VER_STRING_GOES_HERE
  Inverter : xbsIndex_r4/Inverter  en=off  latency=0  dbl_ovrd=off  block_type=inv
      settings: xl_area=[0 0 0 0 0 0 0]  block_version=VER_STRING_GOES_HERE
  Logical1 : xbsIndex_r4/Logical  logical_function=AND  inputs=2  en=off  latency=0  precision=Full  arith_type=Unsigned  n_bits=8  bin_pt=2  align_bp=on  dbl_ovrd=off  block_type=logical
      settings: xl_area=[1 0 0 1 0 0 0]  block_version=VER_STRING_GOES_HERE
  ToBool : xbsIndex_r4/Convert  arith_type=Boolean  n_bits=16  bin_pt=14  quantization=Truncate  overflow=Wrap  latency=0  dbl_ovrd=off  pipeline=off  block_type=convert
      settings: block_version=8.2.02
  Q : Outport
  wires:
  Logical1/1 -> ToBool/1
  D/1 -> Logical1/2, Inverter/1
  Inverter/1 -> Delay/1
  Delay/1 -> Logical1/1
  ToBool/1 -> Q/1

== system ofdm_txrx_mimo_2x/OFDM Rx MIMO/ADC Inputs & Antenna Selection/Antenna Selection/AntA_Gains/S-R Latch ==
  S : Inport
  R : Inport  Port=2
  Constant1 : xbsIndex_r4/Constant  arith_type=Boolean  const=1  n_bits=1  bin_pt=0  explicit_period=off  period=1  equ=P=C  opselect=C  inp2=PCIN>>17  opr=+  inp1=P  carry=CIN  dbl_ovrd=off  block_type=constant
      settings: block_version=VER_STRING_GOES_HERE
  Register2 : xbsIndex_r4/Register  init=0  rst=on  en=on  dbl_ovrd=off  block_type=register
      settings: xl_area=[1 1 0 0 0 0 0]  block_version=VER_STRING_GOES_HERE
  Q : Outport
  wires:
  S/1 -> Register2/3
  Register2/1 -> Q/1
  R/1 -> Register2/2
  Constant1/1 -> Register2/1

== system ofdm_txrx_mimo_2x/OFDM Rx MIMO/ADC Inputs & Antenna Selection/Antenna Selection/AntB_Gains/Posedge1 ==
  note: Rising Edge Detection
  D : Inport
  Delay : xbsIndex_r4/Delay  en=off  latency=1  dbl_ovrd=off  reg_retiming=off  block_type=delay
      settings: infoedit=Hardware notes: A delay line is a chain, each link of which is an SRL16 followed by a flip-flop. If register retiming is enabled, the delay line is a chain of flip-flops.  xl_area=[1 1 0 0 0 0 0]  block_version=VER_STRING_GOES_HERE
  Inverter : xbsIndex_r4/Inverter  en=off  latency=0  dbl_ovrd=off  block_type=inv
      settings: xl_area=[0 0 0 0 0 0 0]  block_version=VER_STRING_GOES_HERE
  Logical1 : xbsIndex_r4/Logical  logical_function=AND  inputs=2  en=off  latency=0  precision=Full  arith_type=Unsigned  n_bits=8  bin_pt=2  align_bp=on  dbl_ovrd=off  block_type=logical
      settings: xl_area=[1 0 0 1 0 0 0]  block_version=VER_STRING_GOES_HERE
  ToBool : xbsIndex_r4/Convert  arith_type=Boolean  n_bits=16  bin_pt=14  quantization=Truncate  overflow=Wrap  latency=0  dbl_ovrd=off  pipeline=off  block_type=convert
      settings: block_version=8.2.02
  Q : Outport
  wires:
  Delay/1 -> Logical1/1
  Inverter/1 -> Delay/1
  D/1 -> Inverter/1, Logical1/2
  Logical1/1 -> ToBool/1
  ToBool/1 -> Q/1

== system ofdm_txrx_mimo_2x/OFDM Rx MIMO/ADC Inputs & Antenna Selection/Antenna Selection/AntB_Gains/S-R Latch1 ==
  S : Inport
  R : Inport  Port=2
  Constant1 : xbsIndex_r4/Constant  arith_type=Boolean  const=1  n_bits=1  bin_pt=0  explicit_period=off  period=1  equ=P=C  opselect=C  inp2=PCIN>>17  opr=+  inp1=P  carry=CIN  dbl_ovrd=off  block_type=constant
      settings: block_version=VER_STRING_GOES_HERE
  Register2 : xbsIndex_r4/Register  init=0  rst=on  en=on  dbl_ovrd=off  block_type=register
      settings: xl_area=[1 1 0 0 0 0 0]  block_version=VER_STRING_GOES_HERE
  Q : Outport
  wires:
  Constant1/1 -> Register2/1
  R/1 -> Register2/2
  Register2/1 -> Q/1
  S/1 -> Register2/3

== system ofdm_txrx_mimo_2x/OFDM Rx MIMO/Coarse Freq Correction/Coarse Freq Offset Calc Combo/Accum Control/8xDebounce ==
  D : Inport
  Delay{1,4..9} (7 alike) : xbsIndex_r4/Delay  Orientation=down  en=off  latency=1  dbl_ovrd=off  reg_retiming=off  block_type=delay
      settings: infoedit=Hardware notes: A delay line is a chain, each link of which is an SRL16 followed by a flip-flop. If register retiming is enabled, the delay line is a chain of flip-flops.  xl_area=[1 1 0 0 0 0 0]  block_version=VER_STRING_GOES_HERE
  Logical5 : xbsIndex_r4/Logical  logical_function=OR  inputs=8  en=off  latency=0  precision=Full  arith_type=Unsigned  n_bits=8  bin_pt=2  align_bp=on  dbl_ovrd=off  block_type=logical
      settings: xl_area=[0, 0, 0, 0, 0, 0, 0]  block_version=VER_STRING_GOES_HERE
  Q : Outport
  wires:
  Delay1/1 -> Delay5/1, Logical5/2
  Logical5/1 -> Q/1
  Delay9/1 -> Logical5/8
  Delay8/1 -> Logical5/7, Delay9/1
  Delay7/1 -> Delay8/1, Logical5/6
  Delay6/1 -> Logical5/5, Delay7/1
  Delay4/1 -> Logical5/4, Delay6/1
  Delay5/1 -> Logical5/3, Delay4/1
  D/1 -> Delay1/1, Logical5/1

== system ofdm_txrx_mimo_2x/OFDM Rx MIMO/Coarse Freq Correction/Coarse Freq Offset Calc Combo/Accum Control/Posedge3 ==
  note: Rising Edge Detection
  D : Inport
  Delay : xbsIndex_r4/Delay  en=off  latency=1  dbl_ovrd=off  reg_retiming=off  block_type=delay
      settings: infoedit=Hardware notes: A delay line is a chain, each link of which is an SRL16 followed by a flip-flop. If register retiming is enabled, the delay line is a chain of flip-flops.  xl_area=[1 1 0 0 0 0 0]  block_version=VER_STRING_GOES_HERE
  Inverter : xbsIndex_r4/Inverter  en=off  latency=0  dbl_ovrd=off  block_type=inv
      settings: xl_area=[0 0 0 0 0 0 0]  block_version=VER_STRING_GOES_HERE
  Logical1 : xbsIndex_r4/Logical  logical_function=AND  inputs=2  en=off  latency=0  precision=Full  arith_type=Unsigned  n_bits=8  bin_pt=2  align_bp=on  dbl_ovrd=off  block_type=logical
      settings: xl_area=[1 0 0 1 0 0 0]  block_version=VER_STRING_GOES_HERE
  Q : Outport
  wires:
  Delay/1 -> Inverter/1
  Inverter/1 -> Logical1/1
  Logical1/1 -> Q/1
  D/1 -> Delay/1, Logical1/2

== system ofdm_txrx_mimo_2x/OFDM Rx MIMO/Coarse Freq Correction/Coarse Freq Offset Calc Combo/Accum Control/S-R Latch1 ==
  R : Inport
  S : Inport  Port=2
  Constant1 : xbsIndex_r4/Constant  arith_type=Boolean  const=1  n_bits=1  bin_pt=0  explicit_period=off  period=1  equ=P=C  opselect=C  inp2=PCIN>>17  opr=+  inp1=P  carry=CIN  dbl_ovrd=off  block_type=constant
      settings: block_version=VER_STRING_GOES_HERE
  Register2 : xbsIndex_r4/Register  init=0  rst=on  en=on  dbl_ovrd=off  block_type=register
      settings: xl_area=[1 1 0 0 0 0 0]  block_version=VER_STRING_GOES_HERE
  Q : Outport
  wires:
  Constant1/1 -> Register2/1
  R/1 -> Register2/2
  Register2/1 -> Q/1
  S/1 -> Register2/3

== system ofdm_txrx_mimo_2x/OFDM Rx MIMO/Coarse Freq Correction/Coarse Freq Offset Calc Combo/Arctan2/CORDIC ATAN1 ==
  note: The CORDIC algorithm is implemented in 3 steps.
      |
      | Step 1: Coarse Angle Rotation.  The algorithm converges only for angles between -pi/2 and pi/2, so if x < zero, the input vector is reflected
      | to the 1st or 3rd quadrant by making the x-coordinate non-negative.
      |
      | Step 2: Fine Angle Rotation.  For rectangular-to-polar conversion, the resulting vector is rotated through progressively smaller angles, such that y goes
      | to zero. In the i-th stage, the angular rotation is by either +/- atan(1/2^i), depending on whether or not its input y is less than or greater than zero.
      |
      | Step 3: Angle Correction. If there was a reflection applied in Step 1, this step applies the appropriate angle correction by subtracting it from +/- pi.
  x : Inport
  y : Inport  Port=2
  CORDIC  Pipe Balance : xbsIndex_r4/Delay  en=off  latency=sum(pipeline)  dbl_ovrd=off  reg_retiming=off  block_type=delay
      settings: infoedit="Delay line having  configurable length.<P><P>Hardware notes: A delay line is a chain, each link of which is an SRL16 followed by a flip-flop. If register retiming is enabled, the delay line is a chain of flip-flops."  xl_area=[0, 0, 0, 0, 0, 0, 0]  block_version=VER_STRING_GOES_HERE
  CORDIC  Pipe Balance1 : xbsIndex_r4/Delay  en=off  latency=sum(pipeline)+1  dbl_ovrd=off  reg_retiming=off  block_type=delay
      settings: infoedit="Delay line having  configurable length.<P><P>Hardware notes: A delay line is a chain, each link of which is an SRL16 followed by a flip-flop. If register retiming is enabled, the delay line is a chain of flip-flops."  xl_area=[0, 0, 0, 0, 0, 0, 0]  block_version=VER_STRING_GOES_HERE
  CORDIC  Pipe Balance2 : xbsIndex_r4/Delay  en=off  latency=1  dbl_ovrd=off  reg_retiming=off  block_type=delay
      settings: infoedit="Delay line having  configurable length.<P><P>Hardware notes: A delay line is a chain, each link of which is an SRL16 followed by a flip-flop. If register retiming is enabled, the delay line is a chain of flip-flops."  xl_area=[0, 0, 0, 0, 0, 0, 0]  block_version=VER_STRING_GOES_HERE
  CORDIC Fine Angle PE : Subsystem  mask(CORDIC parallel PE){}
      mask init:
      | [bg,fg] = xlcmap('XBlock',0);
      | iPos = get_param(gcb,'Position');
      | iWidth=iPos(3)-iPos(1); iHeight=iPos(4)-iPos(2);
      | iCx=iWidth/2;
      | iCy=iHeight/2;
      | [logoX, logoY] = xlogo(iPos);
      mask Display:
      | patch([0 iWidth iWidth 0],[0 0 iHeight iHeight] , bg);
      | patch(logoX,logoY , fg);
      | port_label('input',1,'x');
      | port_label('input',2,'y');
      | port_label('input',3,'z');
      | port_label('output',1,'X');
      | port_label('output',2,'Z');
      | plot([0 0 iWidth iWidth 0], [0 iHeight iHeight 0 0]);
  Constant : xbsIndex_r4/Constant  arith_type=Signed (2's comp)  const=0  n_bits=pe_nbits  bin_pt=pe_binpt  explicit_period=off  period=1  equ=P=C  opselect=C  inp2=PCIN>>17  opr=+  inp1=P  carry=CIN  dbl_ovrd=off  block_type=constant
      settings: block_version=VER_STRING_GOES_HERE
  Quadrant Correct : Subsystem
      mask init:
      | [bg,fg] = xlcmap('XBlock',0);
      | iPos = get_param(gcb,'Position');
      | iWidth=iPos(3)-iPos(1); iHeight=iPos(4)-iPos(2);
      | iCx=iWidth/2;
      | iCy=iHeight/2;
      | [logoX, logoY] = xlogo(iPos);
      mask Display:
      | patch([0 iWidth iWidth 0],[0 0 iHeight iHeight] , bg);
      | patch(logoX,logoY , fg);
      | port_label('input',1,'Z in');
      | port_label('input',2,'sgn(y)');
      | port_label('input',3,'sgn(x)');
      | port_label('output',1,'Z');
      | plot([0 0 iWidth iWidth 0], [0 iHeight iHeight 0 0]);
  Quadrant Map : Subsystem
      mask init:
      | [bg,fg] = xlcmap('XBlock',0);
      | iPos = get_param(gcb,'Position');
      | iWidth=iPos(3)-iPos(1); iHeight=iPos(4)-iPos(2);
      | iCx=iWidth/2;
      | iCy=iHeight/2;
      | [logoX, logoY] = xlogo(iPos);
      mask Display:
      | patch([0 iWidth iWidth 0],[0 0 iHeight iHeight] , bg);
      | patch(logoX,logoY , fg);
      | port_label('input',1,'x');
      | port_label('input',2,'y');
      | port_label('output',1,'X');
      | port_label('output',2,'Y');
      | port_label('output',3,'sgn(y)');
      | port_label('output',4,'sgn(x)');
      | plot([0 0 iWidth iWidth 0], [0 iHeight iHeight 0 0]);
  X : Outport
  Z : Outport  Port=2
  wires:
  Constant/1 -> CORDIC Fine Angle PE/3(z)
  Quadrant Map/1(X) -> CORDIC Fine Angle PE/1(x)
  Quadrant Map/2(Y) -> CORDIC Fine Angle PE/2(y)
  x/1 -> Quadrant Map/1(x)
  y/1 -> Quadrant Map/2(y)
  Quadrant Map/3(sign(y)) -> CORDIC  Pipe Balance/1
  Quadrant Map/4(sign(x)) -> CORDIC  Pipe Balance1/1
  CORDIC  Pipe Balance/1 -> Quadrant Correct/2(PI_ addsub)
  CORDIC  Pipe Balance1/1 -> Quadrant Correct/3(Z MUX SEL)
  CORDIC Fine Angle PE/2(Z) -> Quadrant Correct/1(Z IN)
  Quadrant Correct/1(Z) -> Z/1
  CORDIC Fine Angle PE/1(X) -> CORDIC  Pipe Balance2/1
  CORDIC  Pipe Balance2/1 -> X/1
